simulink library "Renault_Library"  (Simulink 9.2)
settings: Solver=ode3  StartTime=0.0  StopTime=10.0  FixedStep=0.020  RelTol=1e-3  EnableMultiTasking=on
undefined symbols (not defined in the file or in its folder): ABC, AssystemProposal, b, chkval, CoeffA_C, CoeffB_C, CoeffMean_C, ConfTime_C, DiagDelay_C, DividePercent, FailureConfActive_C, FallingEdgeDuration_C, HighLevelThresholdVoltage_C, HighLevelVoltage_C, HighLevelVoltageTunable_C, InitValue_C, InputVoltRangeMax_C, InputVoltRangeMin_C, LowActiveContact_C, LowLevelThresholdVoltage_C, LowLevelVoltage_C, MaxOperatingVoltage_C, MinOperatingVoltage_C, mV2V_conversion, OUT1, OutOfRangeHigh_C, OutOfRangeLow_C, PinSupplySaturationMax, PinSupplySaturationMin, Res1Value_C, Res2Value_C, Res3Value_C, RisingEdgeDuration_C, RSA_CANSignalFailMonitoring, SampleTime_C, service14Table, service19Table, service22Table, service24Table, service28Table, service2ETable, service2FTable, service31Table, service87Table, SizeXStateDID2FTable, SizeXTable14, SizeXTable19, SizeXTable22, SizeXTable24, SizeXTable28, SizeXTable2E, SizeXTable2F, SizeXTable31, SizeXTable87, SizeYStateDID2FTable, SizeYTable14, SizeYTable19, SizeYTable22, SizeYTable24, SizeYTable28 ... (+22)
undefined symbols (not defined in the file): BatteryVoltage_ActivationThreshold_C, FrameStatus_AbsentValue_C, FrameStatus_ConfAbsentValue_C, MemThreshold_C, NAMEOFTHEMODEL_SAMPLE_TIME_CST, NbAbs1_C, NbConfAbs1_C, NbConfPres1_C, NbPres1_C, NOMDUSIGNAL_FaultConfTime_C, NOMDUSIGNAL_FaultRehabTime_C, NOMDUSIGNAL_switchvalue_C, NOMDUSIGNAL_unavailablevalue_C, PeriodFrame1_C, ResetTime_C, WakeUpTime_C
libraries: $bdroot x112, simulink x75
(part 31 of 32)

== stateflow chart Services ECU/Mux/RSA_FrameStatusMgt/FrameMonitoring ==
  state State_Fault_Management_NotAllowed/
    state Frame_present_NA/
      entry:
      Cpt=0;
      during:
      Frame_Status=Frame_Present;
      Cpt=Cpt+Ts;
    state Absent_frame_NA/
      entry: Frame_Status=Frame_Absent_Not_Expected;
    (junction 910) -> Frame_present_NA [order 1] : [Frame_Status==Frame_Present]
    Frame_present_NA -> Frame_present_NA [order 1] : [Frame_Update==1]
    Frame_present_NA -> Absent_frame_NA [order 2] : [(Cpt>=(NbAbs*Period*ToleranceFactor))&&...
        | (Frame_Update==0)]
    Absent_frame_NA -> Frame_present_NA : [Frame_Update==1]
    (default) -> (junction 910)
    (junction 910) -> Absent_frame_NA [order 2] : [Frame_Status~=Frame_Present]
  state State_Fault_Management_Allowed/
    state Frame_present_A/
      entry:
      Cpt=0;
      Frame_Status=Frame_Present;
      during:
      Cpt=Cpt+Ts;
    state Fault_TobeForget_A/
      entry:
      Cpt=0;
      during:
      Cpt=Cpt+Ts;
      Cpt2=Cpt2+Ts;
    state Absent_frame_A/
      entry:
      Frame_Status=Frame_Absent;
      during:
      Cpt=Cpt+Ts;
    state Absent_frame_confirmed_A/
      entry: Cpt2=0;
      Frame_Status=Frame_Confirmed_Absent;
    Frame_present_A -> Frame_present_A [order 1] : [Frame_Update==1]
    Fault_TobeForget_A -> Fault_TobeForget_A [order 1] : [(Frame_Update==1)&&...
        | (Cpt2<NbConfPres*Period*...
        | ToleranceFactor)]
    (default) -> (junction 917)
    (junction 917) -> Frame_present_A [order 1] : [Frame_Status==Frame_Present]
    (junction 917) -> Absent_frame_A [order 2] : [Frame_Status~=Frame_Present]
    Fault_TobeForget_A -> Frame_present_A [order 3] : [(Cpt2>=NbConfPres*Period*ToleranceFactor)]
    Absent_frame_confirmed_A -> Frame_present_A [order 1] : [(Frame_Update==1)&&...
        | NbConfPres==0]
    Frame_present_A -> Absent_frame_A [order 2] : [(Cpt>=(NbAbs*Period*...
        | ToleranceFactor))&&...
        | (Frame_Update==0)]
    Absent_frame_A -> Frame_present_A [order 1] : [Frame_Update==1]
    Fault_TobeForget_A -> Absent_frame_confirmed_A [order 2] : [Cpt>=(NbPres*Period*ToleranceFactor)&&...
        | Frame_Update==0&&NbPres~=0]
    Absent_frame_confirmed_A -> Fault_TobeForget_A [order 2] : [Frame_Update==1&&NbConfPres~=0]
    Absent_frame_A -> Absent_frame_confirmed_A [order 2] : [(Cpt>=(NbConfAbs*Period*ToleranceFactor))&&(Frame_Update==0)]
  (default) -> State_Fault_Management_NotAllowed
  State_Fault_Management_NotAllowed -> State_Fault_Management_Allowed : [ Fault_Management_Allowed == 1 ]
  State_Fault_Management_Allowed -> State_Fault_Management_NotAllowed : [ Fault_Management_Allowed == 0 && ...
      | Frame_Status ~= Frame_Confirmed_Absent ]
  data: Fault_Management_Allowed input boolean; Frame_Update input uint8; Ts input double =Ts; Frame_Status output double =1; Cpt output double; Period input double; NbAbs input double; NbConfAbs input double; NbPres input double; Frame_Absent parameter double; Frame_Absent_Not_Expected parameter double; Frame_Confirmed_Absent parameter double; Frame_Present parameter double; NbConfPres input double; ToleranceFactor parameter double; Cpt2 output double

== stateflow chart Services ECU/PowerSupply/RSA_PowerSupplyMgt/PowerSupMgt_Chart ==
  state Ecu_enabled/
    state WakeUp/
      entry:
      ActivateEcu=Ecu_ON;
      EcuStatus=Status_WAKEUP;
      read = 1;
      write = 0;
    state WithoutInit/
      entry: EcuStatus=Status_ON;
      read = 1;
      write = 0;
    state Ecu_Running/
      entry: EcuStatus=Status_ON;
      read = 0;
      write = 0;
    (default) -> WakeUp [order 1] : [WakeUpTime~=0]
    (default) -> WithoutInit [order 2] : [WakeUpTime==0]
    WakeUp -> Ecu_Running : [after(WakeUpTime/SampleTime,...
        | tick)]
    WithoutInit -> Ecu_Running
  state Ecu_waiting_apc/
    entry: ActivateEcu=Ecu_OFF;
    EcuStatus = Status_OFF;
    read=0;
    write=0;
  state WithoutReset/
    entry: EcuStatus=Status_RESET;
    read = 0;
    write = 1;
  state Reset/
    entry:
    EcuStatus=Status_RESET;
    read = 0;
    write = 1;
  (default) -> Ecu_waiting_apc
  Ecu_waiting_apc -> Ecu_enabled : [Apc==Apc_ON &&...
      | BatteryVoltage>=...
      | BatteryVoltageThreshold]
  Ecu_enabled -> Ecu_waiting_apc [order 2] : [BatteryVoltage<VoltageSupplyCutThreshold]
  Reset -> Ecu_waiting_apc : [after(ResetTime/SampleTime,tick) ||...
      | BatteryVoltage<...
      | VoltageSupplyCutThreshold]
  WithoutReset -> Ecu_waiting_apc
  Ecu_enabled -> WithoutReset [order 3] : [(Apc==Apc_OFF ||...
      | BatteryVoltage<...
      | BatteryVoltageThreshold) &&...
      | ResetTime==0 &&...
      | BatteryVoltage>...
      | VoltageSupplyCutThreshold]
  Ecu_enabled -> Reset [order 1] : [(Apc==Apc_OFF ||...
      | BatteryVoltage<...
      | BatteryVoltageThreshold) &&...
      | ResetTime~=0 &&...
      | BatteryVoltage>...
      | VoltageSupplyCutThreshold]
  data: ActivateEcu output double; Apc input double; BatteryVoltage input double; BatteryVoltageThreshold input double; VoltageSupplyCutThreshold input double; EcuStatus output uint8; read output boolean; WakeUpTime input double; write output boolean; ResetTime input double; Apc_ON parameter double; Apc_OFF parameter double; Ecu_OFF parameter double; Ecu_ON parameter double; Status_OFF parameter double; Status_WAKEUP parameter double; Status_ON parameter double; Status_RESET parameter double; SampleTime input double

== stateflow chart Services ECU/Diag/RSA_DiagnosticManagement_v02.00/DIAGNOSTIC_SANCTION_TOP/Service24/Check_Message_Service24 ==
  (default) -> (junction 996)
  (junction 996) -> (junction 997) [order 1] : [LENGTH_MESSAGE_CORRECT]
  (junction 997) -> (junction 998) [order 1] : [PARAMETER_VALID]
  (junction 998) -> (junction 999) [order 1] : [SECURITY_ACCESS_ACCEPTED]
  (junction 999) -> (junction 1000) [order 1] : [CONDITION_CORRECT]
  (junction 996) -> (junction 1005) [order 2] : {
      | NEGATIVE_RES_CODE= 0x13 ;
      | }
  (junction 997) -> (junction 1001) [order 2] : {
      | NEGATIVE_RES_CODE= 0x31 ;
      | }
  (junction 998) -> (junction 1002) [order 2] : {
      | NEGATIVE_RES_CODE= 0x33 ;
      | }
  (junction 999) -> (junction 1003) [order 2] : {
      | NEGATIVE_RES_CODE= 0x22 ;
      | }
  (junction 1000) -> (junction 1004)
  (junction 1002) -> (junction 1001)
  (junction 1003) -> (junction 1002)
  (junction 1004) -> (junction 1003)
  (junction 1001) -> (junction 1005)
  (junction 1005) -> (junction 1006) : {
      |  SEND_RES_MESSAGE=!SEND_RES_MESSAGE;
      | }
  data: LENGTH_MESSAGE_CORRECT input boolean; CONDITION_CORRECT input boolean; NEGATIVE_RES_CODE output uint8; SECURITY_ACCESS_ACCEPTED input boolean; PARAMETER_VALID input boolean; SEND_RES_MESSAGE output boolean

== stateflow chart Services ECU/Diag/RSA_DiagnosticManagement_v02.00/DIAGNOSTIC_SANCTION_TOP/Service24/Parameter_Valid_Service24/Parameter_service24 ==
  state Session [function]
    (default) -> (junction 1025)
    (junction 1025) -> (junction 1026) [order 1] : [SESSION_ACTIVE==DEFAULT_SESSION]
        | {
        |  s=1;
        | }
    (junction 1025) -> (junction 1027) [order 2]
    (junction 1026) -> (junction 1028)
    (junction 1027) -> (junction 1028) [order 1] : [SESSION_ACTIVE==PROGRAMMING_SESSION]
        | {
        |  s=2;
        | }
    (junction 1027) -> (junction 1029) [order 2]
    (junction 1028) -> (junction 1030)
    (junction 1029) -> (junction 1030) [order 1] : [SESSION_ACTIVE==EXTENDED_SESSION]
        | {
        |  s=3;
        | }
    (junction 1029) -> (junction 1031) [order 2]
    (junction 1030) -> (junction 1032)
    (junction 1031) -> (junction 1032) [order 1] : [SESSION_ACTIVE==SAFETY_SYSTEM_SESSION]
        | {
        |  s=4;
        | }
    (junction 1031) -> (junction 1033) [order 2]
    (junction 1032) -> (junction 1034)
    (junction 1034) -> (junction 1033)
    (junction 1033) -> (junction 1035)
  (default) -> (junction 1061)
  (junction 1061) -> (junction 1064) : {
      |  i=0;...
      |  SECURITY_ACCESS_ACCEPTED=true;...
      |  PARAMETER_VALID= false;...
      |  DID=(DIAG_BUFFER_REQUEST[1]<<8) + DIAG_BUFFER_REQUEST[2];
      | }
  (junction 1062) -> (junction 1063) [order 1] : [DID == service24Table[i][0] &&...
      |  service24Table[i][s]==1]
  (junction 1064) -> (junction 1062) [order 1] : [i<SizeXTable24]
  (junction 1062) -> (junction 1065) [order 2]
  (junction 1063) -> (junction 1067) : {
      |  PARAMETER_VALID= true;
      | }
  (junction 1065) -> (junction 1064) : {
      |  i ++;
      | }
  (junction 1064) -> (junction 1066) [order 2]
  (junction 1067) -> (junction 1066)
  (junction 1066) -> (junction 1068)
  (junction 1068) -> (junction 1069) [order 1] : [service24Table[i][5]==0]
  (junction 1068) -> (junction 1070) [order 2]
  (junction 1069) -> (junction 1071) : {
      |  PARAMETER_VALID=false;
      | }
  (junction 1071) -> (junction 1070)
  (junction 1070) -> (junction 1072)
  data: DIAG_BUFFER_REQUEST input uint8 size=4095; PARAMETER_VALID output boolean; i local uint32; service24Table parameter double size=[SizeXTable24 SizeYTable24]; SizeYTable24 parameter double; SizeXTable24 parameter double; SECURITY_ACCESS_ACCEPTED output boolean; SESSION_ACTIVE input Enum: TYP_SESSION_ACTIVE; s local double; DID local uint16

== stateflow chart Services ECU/Diag/RSA_DiagnosticManagement_v02.00/DIAGNOSTIC_SANCTION_TOP/Service27/Check_Message_Service27 ==
  (default) -> (junction 1105)
  (junction 1099) -> (junction 1100) [order 1] : [SUBFUNCTION_SUPPORTED_IN_ACTIVE_SESSION]
  (junction 1106) -> (junction 1099) [order 1] : [SUBFUNCTION_SUPPORTED]
  (junction 1100) -> (junction 1101) [order 1] : [PARAMETER_VALID]
  (junction 1101) -> (junction 1102) [order 1] : [CONDITION_CORRECT]
  (junction 1102) -> (junction 1103) [order 1] : [REQ_SEQUENCE_OK]
  (junction 1103) -> (junction 1104) [order 1] : [KEY_VALID]
  (junction 1105) -> (junction 1106) [order 1] : [LENGTH_MESSAGE_CORRECT]
  (junction 1099) -> (junction 1109) [order 2] : {
      | NEGATIVE_RES_CODE= 0x7E ;
      | }
  (junction 1100) -> (junction 1110) [order 2] : {
      | NEGATIVE_RES_CODE= 0x31 ;
      | }
  (junction 1101) -> (junction 1111) [order 2] : {
      | NEGATIVE_RES_CODE= 0x22 ;
      | }
  (junction 1102) -> (junction 1112) [order 2] : {
      | NEGATIVE_RES_CODE= 0x24 ;
      | }
  (junction 1103) -> (junction 1113) [order 2] : {
      | NEGATIVE_RES_CODE= 0x35 ;
      | }
  (junction 1104) -> (junction 1114)
  (junction 1105) -> (junction 1107) [order 2] : {
      | NEGATIVE_RES_CODE= 0x13 ;
      | }
  (junction 1106) -> (junction 1108) [order 2] : {
      | NEGATIVE_RES_CODE= 0x12 ;
      | }
  (junction 1108) -> (junction 1107)
  (junction 1109) -> (junction 1108)
  (junction 1110) -> (junction 1109)
  (junction 1111) -> (junction 1110)
  (junction 1112) -> (junction 1111)
  (junction 1113) -> (junction 1112)
  (junction 1114) -> (junction 1113)
  (junction 1107) -> (junction 1115) : {
      |  SEND_RES_MESSAGE=!SEND_RES_MESSAGE;
      | }
  data: LENGTH_MESSAGE_CORRECT input boolean; CONDITION_CORRECT input boolean; REQ_SEQUENCE_OK input boolean; KEY_VALID input double; NEGATIVE_RES_CODE output uint8; PARAMETER_VALID input boolean; SUBFUNCTION_SUPPORTED_IN_ACTIVE_SESSION input boolean; SUBFUNCTION_SUPPORTED input boolean; SEND_RES_MESSAGE output boolean

== stateflow chart Services ECU/Diag/RSA_DiagnosticManagement_v02.00/DIAGNOSTIC_SANCTION_TOP/Service28/Check_Message_Service28 ==
  (default) -> (junction 1153)
  (junction 1149) -> (junction 1150) [order 1] : [SUBFUNCTION_SUPPORTED_IN_ACTIVE_SESSION]
  (junction 1154) -> (junction 1149) [order 1] : [SUBFUNCTION_SUPPORTED]
  (junction 1150) -> (junction 1151) [order 1] : [PARAMETER_VALID]
  (junction 1151) -> (junction 1152) [order 1] : [CONDITION_CORRECT]
  (junction 1153) -> (junction 1154) [order 1] : [LENGTH_MESSAGE_CORRECT]
  (junction 1149) -> (junction 1157) [order 2] : {
      | NEGATIVE_RES_CODE= 0x7E ;
      | }
  (junction 1150) -> (junction 1158) [order 2] : {
      | NEGATIVE_RES_CODE= 0x31 ;
      | }
  (junction 1151) -> (junction 1159) [order 2] : {
      | NEGATIVE_RES_CODE= 0x22 ;
      | }
  (junction 1152) -> (junction 1160)
  (junction 1153) -> (junction 1155) [order 2] : {
      | NEGATIVE_RES_CODE= 0x13 ;
      | }
  (junction 1154) -> (junction 1156) [order 2] : {
      | NEGATIVE_RES_CODE= 0x12 ;
      | }
  (junction 1156) -> (junction 1155)
  (junction 1157) -> (junction 1156)
  (junction 1158) -> (junction 1157)
  (junction 1159) -> (junction 1158)
  (junction 1160) -> (junction 1159)
  (junction 1155) -> (junction 1161) : {
      |  SEND_RES_MESSAGE=!SEND_RES_MESSAGE;
      | }
  data: LENGTH_MESSAGE_CORRECT input boolean; CONDITION_CORRECT input boolean; NEGATIVE_RES_CODE output uint8; SUBFUNCTION_SUPPORTED_IN_ACTIVE_SESSION input boolean; PARAMETER_VALID input boolean; SUBFUNCTION_SUPPORTED input boolean; SEND_RES_MESSAGE output boolean

== stateflow chart Services ECU/Diag/RSA_DiagnosticManagement_v02.00/DIAGNOSTIC_SANCTION_TOP/Service28/Parameter_Valid_Service28/Parameter_service28 ==
  (default) -> (junction 1189)
  (junction 1189) -> (junction 1192) : {
      |  i=0;...
      |  PARAMETER_VALID= false;...
      |  nodeIdentifierData=(DIAG_BUFFER_REQUEST[3]<<8) + DIAG_BUFFER_REQUEST[4];
      | }
  (junction 1190) -> (junction 1191) [order 1] : [nodeIdentifierData == service28Table[i][0]]
  (junction 1192) -> (junction 1190) [order 1] : [i< SizeXTable28]
  (junction 1190) -> (junction 1193) [order 2]
  (junction 1191) -> (junction 1194) : {
      |  PARAMETER_VALID= true;...
      |
      | }
  (junction 1193) -> (junction 1192) : {
      |  i ++;
      | }
  (junction 1192) -> (junction 1195) [order 2]
  (junction 1194) -> (junction 1193)
  data: nodeIdentifierData local uint16; DIAG_BUFFER_REQUEST input uint8 size=4095; PARAMETER_VALID output boolean; i local double; SizeYTable28 parameter double; SizeXTable28 parameter double; service28Table parameter double size=[SizeXTable28 SizeYTable28]

== stateflow chart Services ECU/Diag/RSA_DiagnosticManagement_v02.00/DIAGNOSTIC_SANCTION_TOP/Service2E/Check_Message_Service2E ==
  (default) -> (junction 1214)
  (junction 1214) -> (junction 1215) [order 1] : [LENGTH_MESSAGE_CORRECT]
  (junction 1215) -> (junction 1216) [order 1] : [PARAMETER_VALID]
  (junction 1216) -> (junction 1217) [order 1] : [SECURITY_ACCESS_ACCEPTED]
  (junction 1217) -> (junction 1218) [order 1] : [CONDITION_CORRECT]
  (junction 1218) -> (junction 1219) [order 1] : [CORRECTLY_ALTERED_INTO_MEMORY]
  (junction 1214) -> (junction 1222) [order 2] : {
      | NEGATIVE_RES_CODE= 0x13 ;
      | }
  (junction 1215) -> (junction 1223) [order 2] : {
      | NEGATIVE_RES_CODE= 0x31 ;
      | }
  (junction 1216) -> (junction 1224) [order 2] : {
      | NEGATIVE_RES_CODE= 0x33 ;
      | }
  (junction 1217) -> (junction 1225) [order 2] : {
      | NEGATIVE_RES_CODE= 0x22 ;
      | }
  (junction 1218) -> (junction 1220) [order 2] : {
      | NEGATIVE_RES_CODE= 0x72 ;
      | }
  (junction 1219) -> (junction 1221)
  (junction 1221) -> (junction 1220)
  (junction 1223) -> (junction 1222)
  (junction 1220) -> (junction 1225)
  (junction 1224) -> (junction 1223)
  (junction 1225) -> (junction 1224)
  (junction 1222) -> (junction 1226) : {
      |  SEND_RES_MESSAGE=!SEND_RES_MESSAGE;
      | }
  data: LENGTH_MESSAGE_CORRECT input boolean; CONDITION_CORRECT input boolean; CORRECTLY_ALTERED_INTO_MEMORY input boolean; NEGATIVE_RES_CODE output uint8; SECURITY_ACCESS_ACCEPTED input boolean; PARAMETER_VALID input boolean; SEND_RES_MESSAGE output boolean

== stateflow chart Services ECU/Diag/RSA_DiagnosticManagement_v02.00/DIAGNOSTIC_SANCTION_TOP/Service2E/Parameter_Valid_Service2E/Parameter_service2E ==
  state Session [function]
    (default) -> (junction 1248)
    (junction 1248) -> (junction 1249) [order 1] : [SESSION_ACTIVE==DEFAULT_SESSION]
        | {
        |  s=1;
        | }
    (junction 1248) -> (junction 1250) [order 2]
    (junction 1249) -> (junction 1251)
    (junction 1250) -> (junction 1251) [order 1] : [SESSION_ACTIVE==PROGRAMMING_SESSION]
        | {
        |  s=2;
        | }
    (junction 1250) -> (junction 1252) [order 2]
    (junction 1251) -> (junction 1253)
    (junction 1252) -> (junction 1253) [order 1] : [SESSION_ACTIVE==EXTENDED_SESSION]
        | {
        |  s=3;
        | }
    (junction 1252) -> (junction 1254) [order 2]
    (junction 1253) -> (junction 1255)
    (junction 1254) -> (junction 1255) [order 1] : [SESSION_ACTIVE==SAFETY_SYSTEM_SESSION]
        | {
        |  s=4;
        | }
    (junction 1254) -> (junction 1256) [order 2]
    (junction 1255) -> (junction 1257)
    (junction 1257) -> (junction 1256)
    (junction 1256) -> (junction 1258)
  (default) -> (junction 1288) : {
      |  j=0;...
      |  SECURITY_ACCESS_ACCEPTED=true;...
      |  PARAMETER_VALID= false;...
      |  number_bit_data=0;
      | }
  (junction 1286) -> (junction 1287) [order 1] : [DID == service2ETable[j][0] &&...
      |  service2ETable[j][s]==1  ]
  (junction 1288) -> (junction 1286) [order 1] : [j< SizeXTable2E ]
      | {
      | DID=(DIAG_BUFFER_REQUEST[1]<<8) + DIAG_BUFFER_REQUEST[2];...
      | Session();
      | }
  (junction 1286) -> (junction 1291) [order 2]
  (junction 1287) -> (junction 1289) : {
      |  number_bit_data+=service2ETable[j][6];
      | }
  (junction 1291) -> (junction 1288) : {
      |  j ++;
      | }
  (junction 1288) -> (junction 1297) [order 2]
  (junction 1289) -> (junction 1290) [order 1] : [uint8 ((number_bit_data+7)/8)==(REQ_DATA_NUMBER-3)]
  (junction 1289) -> (junction 1292) [order 2]
  (junction 1290) -> (junction 1293) : {
      |  PARAMETER_VALID= true;
      | }
  (junction 1292) -> (junction 1291)
  (junction 1295) -> (junction 1292)
  (junction 1293) -> (junction 1294) [order 1] : [service2ETable[j][5]==0]
  (junction 1293) -> (junction 1295) [order 2]
  (junction 1294) -> (junction 1296) : {
      |  SECURITY_ACCESS_ACCEPTED=false;
      | }
  (junction 1296) -> (junction 1295)
  data: DIAG_BUFFER_REQUEST input uint8 size=4095; REQ_DATA_NUMBER input uint8; PARAMETER_VALID output boolean; DID local uint16; j local uint32; service2ETable parameter double size=[SizeXTable2E SizeYTable2E]; SizeYTable2E parameter double; SizeXTable2E parameter double; SECURITY_ACCESS_ACCEPTED output boolean; SESSION_ACTIVE input Enum: TYP_SESSION_ACTIVE; s local double; number_bit_data local double

== stateflow chart Services ECU/Diag/RSA_DiagnosticManagement_v02.00/DIAGNOSTIC_SANCTION_TOP/Service2F/Check_Message_Service2F ==
  (default) -> (junction 1323)
  (junction 1323) -> (junction 1322) [order 1] : [LENGTH_MESSAGE_CORRECT]
  (junction 1322) -> (junction 1324) [order 1] : [CONDITION_CORRECT]
  (junction 1324) -> (junction 1325) [order 1] : [PARAMETER_VALID]
  (junction 1325) -> (junction 1326) [order 1] : [SECURITY_ACCESS_ACCEPTED]
  (junction 1322) -> (junction 1327) [order 2] : {
      | NEGATIVE_RES_CODE= 0x22 ;
      | }
  (junction 1323) -> (junction 1328) [order 2] : {
      | NEGATIVE_RES_CODE= 0x13 ;
      | }
  (junction 1324) -> (junction 1329) [order 2] : {
      | NEGATIVE_RES_CODE= 0x31;
      | }
  (junction 1325) -> (junction 1330) [order 2] : {
      | NEGATIVE_RES_CODE= 0x33
      | }
  (junction 1326) -> (junction 1331)
  (junction 1329) -> (junction 1327)
  (junction 1327) -> (junction 1328)
  (junction 1330) -> (junction 1329)
  (junction 1331) -> (junction 1330)
  (junction 1328) -> (junction 1332) : {
      |  SEND_RES_MESSAGE=!SEND_RES_MESSAGE;
      | }
  data: LENGTH_MESSAGE_CORRECT input boolean; CONDITION_CORRECT input boolean; NEGATIVE_RES_CODE output uint8; SECURITY_ACCESS_ACCEPTED input boolean; PARAMETER_VALID input boolean; SEND_RES_MESSAGE output boolean

== stateflow chart Services ECU/Diag/RSA_DiagnosticManagement_v02.00/DIAGNOSTIC_SANCTION_TOP/Service2F/Parameter_Valid_Service2F/Parameter_service2F ==
  state Session [function]
    (default) -> (junction 1351)
    (junction 1351) -> (junction 1352) [order 1] : [SESSION_ACTIVE==DEFAULT_SESSION]
        | {
        |  s=1;
        | }
    (junction 1351) -> (junction 1353) [order 2]
    (junction 1352) -> (junction 1354)
    (junction 1353) -> (junction 1354) [order 1] : [SESSION_ACTIVE==PROGRAMMING_SESSION]
        | {
        |  s=2;
        | }
    (junction 1353) -> (junction 1355) [order 2]
    (junction 1354) -> (junction 1356)
    (junction 1355) -> (junction 1356) [order 1] : [SESSION_ACTIVE==EXTENDED_SESSION]
        | {
        |  s=3;
        | }
    (junction 1355) -> (junction 1357) [order 2]
    (junction 1356) -> (junction 1358)
    (junction 1357) -> (junction 1358) [order 1] : [SESSION_ACTIVE==SAFETY_SYSTEM_SESSION]
        | {
        |  s=4;
        | }
    (junction 1357) -> (junction 1359) [order 2]
    (junction 1358) -> (junction 1360)
    (junction 1360) -> (junction 1359)
    (junction 1359) -> (junction 1361)
  (default) -> (junction 1391)
  (junction 1391) -> (junction 1397) : {
      |  i=0;
      |  j=0;...
      |  PARAMETER_VALID= false;...
      |  DID=(DIAG_BUFFER_REQUEST[1]<<8) + DIAG_BUFFER_REQUEST[2];
      | }
  (junction 1392) -> (junction 1393) [order 1] : [DID== stateDID2FTable[i][0] &&...
      |         DIAG_BUFFER_REQUEST[4]==stateDID2FTable[i][1]]
  (junction 1396) -> (junction 1392) [order 1] : [i<SizeXStateDID2FTable]
  (junction 1392) -> (junction 1400) [order 2]
  (junction 1393) -> (junction 1401) : {
      |  PARAMETER_VALID= true;...
      | }
  (junction 1394) -> (junction 1395) [order 1] : [DID== service2FTable[j][0] &&...
      |         service2FTable[j][s]==1]
  (junction 1395) -> (junction 1396) [order 1] : [DIAG_BUFFER_REQUEST[3]== 3 &&...
      |         service2FTable[j][9]==1 ]
  (junction 1397) -> (junction 1394) [order 1] : [j< SizeXTable2F]
      | {
      |  Session();
      | }
  (junction 1400) -> (junction 1396) : {
      | i++;
      | }
  (junction 1394) -> (junction 1404) [order 2]
  (junction 1395) -> (junction 1398) [order 2]
  (junction 1396) -> (junction 1408) [order 2]
  (junction 1404) -> (junction 1397) : {
      |  j ++;
      | }
  (junction 1397) -> (junction 1411) [order 2]
  (junction 1398) -> (junction 1399) [order 1] : [DIAG_BUFFER_REQUEST[3]== 2 &&...
      |         service2FTable[j][8]==1]
  (junction 1398) -> (junction 1402) [order 2]
  (junction 1399) -> (junction 1407) : {
      |  PARAMETER_VALID= true;...
      | }
  (junction 1401) -> (junction 1400)
  (junction 1402) -> (junction 1403) [order 1] : [DIAG_BUFFER_REQUEST[3]== 1 &&...
      |         service2FTable[j][7]==1]
  (junction 1402) -> (junction 1405) [order 2]
  (junction 1403) -> (junction 1406) : {
      |  PARAMETER_VALID= true;...
      | }
  (junction 1416) -> (junction 1404)
  (junction 1406) -> (junction 1405)
  (junction 1407) -> (junction 1406)
  (junction 1408) -> (junction 1407)
  (junction 1405) -> (junction 1409)
  (junction 1409) -> (junction 1410) [order 1] : [DIAG_BUFFER_REQUEST[3]== 0 &&...
      |         service2FTable[j][6]==1]
  (junction 1409) -> (junction 1412) [order 2]
  (junction 1410) -> (junction 1413) : {
      |  PARAMETER_VALID= true;...
      | }
  (junction 1413) -> (junction 1412)
  (junction 1412) -> (junction 1414)
  (junction 1414) -> (junction 1415) [order 1] : [service2FTable[j][5]==1]
  (junction 1414) -> (junction 1416) [order 2]
  (junction 1415) -> (junction 1417) : {
      |  SECURITY_ACCESS_ACCEPTED=true;
      | }
  (junction 1417) -> (junction 1416)
  data: SESSION_ACTIVE input Enum: TYP_SESSION_ACTIVE; SECURITY_ACCESS_ACCEPTED output double; DIAG_BUFFER_REQUEST input uint8 size=4095; PARAMETER_VALID output boolean; DID local uint16; j local uint32; service2FTable parameter double size=[SizeXTable2F SizeYTable2F]; s local double; SizeYTable2F parameter double; stateDID2FTable parameter double size=[SizeXStateDID2FTable SizeYStateDID2FTable]; SizeXTable2F parameter double; SizeXStateDID2FTable parameter double; SizeYStateDID2FTable parameter double; i local double

== stateflow chart Services ECU/Diag/RSA_DiagnosticManagement_v02.00/DIAGNOSTIC_SANCTION_TOP/Service31/Check_Message_Service31 ==
  (default) -> (junction 1471)
  (junction 1472) -> (junction 1465) [order 1] : [SUBFUNCTION_SUPPORTED]
  (junction 1465) -> (junction 1466) [order 1] : [SUBFUNCTION_SUPPORTED_IN_ACTIVE_SESSION]
  (junction 1466) -> (junction 1467) [order 1] : [PARAMETER_VALID]
  (junction 1467) -> (junction 1468) [order 1] : [SECURITY_ACCESS_ACCEPTED]
  (junction 1468) -> (junction 1469) [order 1] : [CONDITION_CORRECT]
  (junction 1469) -> (junction 1470) [order 1] : [REQ_SEQUENCE_OK]
  (junction 1471) -> (junction 1472) [order 1] : [LENGTH_MESSAGE_CORRECT]
  (junction 1465) -> (junction 1478) [order 2] : {
      | NEGATIVE_RES_CODE= 0x7E ;
      | }
  (junction 1466) -> (junction 1479) [order 2] : {
      | NEGATIVE_RES_CODE= 0x31
      | }
  (junction 1467) -> (junction 1480) [order 2] : {
      | NEGATIVE_RES_CODE= 0x33 ;
      | }
  (junction 1468) -> (junction 1474) [order 2] : {
      | NEGATIVE_RES_CODE= 0x22 ;
      | }
  (junction 1469) -> (junction 1475) [order 2] : {
      | NEGATIVE_RES_CODE= 0x24 ;
      | }
  (junction 1470) -> (junction 1476)
  (junction 1471) -> (junction 1473) [order 2] : {
      | NEGATIVE_RES_CODE= 0x13 ;
      | }
  (junction 1472) -> (junction 1477) [order 2] : {
      | NEGATIVE_RES_CODE= 0x12 ;
      | }
  (junction 1477) -> (junction 1473)
  (junction 1475) -> (junction 1474)
  (junction 1476) -> (junction 1475)
  (junction 1474) -> (junction 1480)
  (junction 1478) -> (junction 1477)
  (junction 1479) -> (junction 1478)
  (junction 1480) -> (junction 1479)
  (junction 1473) -> (junction 1481) : {
      |  SEND_RES_MESSAGE=!SEND_RES_MESSAGE;
      | }
  data: LENGTH_MESSAGE_CORRECT input boolean; REQ_SEQUENCE_OK input boolean; NEGATIVE_RES_CODE output uint8; CONDITION_CORRECT input boolean; SECURITY_ACCESS_ACCEPTED input boolean; PARAMETER_VALID input double; SUBFUNCTION_SUPPORTED_IN_ACTIVE_SESSION input boolean; SUBFUNCTION_SUPPORTED input boolean; SEND_RES_MESSAGE output boolean

== stateflow chart Services ECU/Diag/RSA_DiagnosticManagement/DIAGNOSTIC_SANCTION_TOP/Service10/Check_Message_Service10 ==
  (default) -> (junction 1513)
  (junction 1513) -> (junction 1514) [order 1] : [LENGTH_MESSAGE_CORRECT]
  (junction 1514) -> (junction 1515) [order 1] : [SUBFUNCTION_SUPPORTED]
  (junction 1515) -> (junction 1516) [order 1] : [CONDITION_CORRECT]
  (junction 1513) -> (junction 1517) [order 2] : {
      | NEGATIVE_RES_CODE= 0x13 ;
      | }
  (junction 1514) -> (junction 1518) [order 2] : {
      | NEGATIVE_RES_CODE= 0x12 ;
      | }
  (junction 1515) -> (junction 1519) [order 2] : {
      | NEGATIVE_RES_CODE= 0x22 ;
      | }
  (junction 1516) -> (junction 1520)
  (junction 1518) -> (junction 1517)
  (junction 1519) -> (junction 1518)
  (junction 1520) -> (junction 1519)
  (junction 1517) -> (junction 1521) : {
      |  SEND_RES_MESSAGE=!SEND_RES_MESSAGE;
      | }
  data: SUBFUNCTION_SUPPORTED input boolean; LENGTH_MESSAGE_CORRECT input boolean; CONDITION_CORRECT input boolean; NEGATIVE_RES_CODE output uint8; SEND_RES_MESSAGE output boolean

== stateflow chart Services ECU/Diag/RSA_DiagnosticManagement/DIAGNOSTIC_SANCTION_TOP/Service11/Check_Message_Service11 ==
  (default) -> (junction 1548)
  (junction 1543) -> (junction 1544) [order 1] : [SUBFUNCTION_SUPPORTED_IN_ACTIVE_SESSION]
  (junction 1544) -> (junction 1545) [order 1] : [SECURITY_ACCESS_ACCEPTED]
  (junction 1545) -> (junction 1546) [order 1] : [CONDITION_CORRECT]
  (junction 1547) -> (junction 1543) [order 1] : [SUBFUNCTION_SUPPORTED]
  (junction 1548) -> (junction 1547) [order 1] : [LENGTH_MESSAGE_CORRECT]
  (junction 1543) -> (junction 1551) [order 2] : {
      | NEGATIVE_RES_CODE= 0x7E ;
      | }
  (junction 1544) -> (junction 1552) [order 2] : {
      | NEGATIVE_RES_CODE= 0x33 ;
      | }
  (junction 1545) -> (junction 1553) [order 2] : {
      | NEGATIVE_RES_CODE= 0x22 ;
      | }
  (junction 1546) -> (junction 1554)
  (junction 1547) -> (junction 1550) [order 2] : {
      | NEGATIVE_RES_CODE= 0x12 ;
      | }
  (junction 1548) -> (junction 1549) [order 2] : {
      | NEGATIVE_RES_CODE= 0x13 ;
      | }
  (junction 1550) -> (junction 1549)
  (junction 1551) -> (junction 1550)
  (junction 1552) -> (junction 1551)
  (junction 1553) -> (junction 1552)
  (junction 1554) -> (junction 1553)
  (junction 1549) -> (junction 1555) : {
      |  SEND_RES_MESSAGE=!SEND_RES_MESSAGE;
      | }
  data: SUBFUNCTION_SUPPORTED input boolean; SUBFUNCTION_SUPPORTED_IN_ACTIVE_SESSION input boolean; LENGTH_MESSAGE_CORRECT input boolean; CONDITION_CORRECT input boolean; NEGATIVE_RES_CODE output uint8; SECURITY_ACCESS_ACCEPTED input boolean; SEND_RES_MESSAGE output boolean

== stateflow chart Services ECU/Diag/RSA_DiagnosticManagement/DIAGNOSTIC_SANCTION_TOP/Service14/Check_Message_Service14 ==
  (default) -> (junction 1584)
  (junction 1581) -> (junction 1582) [order 1] : [PARAMETER_VALID]
  (junction 1582) -> (junction 1583) [order 1] : [CONDITION_CORRECT]
  (junction 1584) -> (junction 1581) [order 1] : [LENGTH_MESSAGE_CORRECT]
  (junction 1581) -> (junction 1585) [order 2] : {
      | NEGATIVE_RES_CODE= 0x31 ;
      | }
  (junction 1582) -> (junction 1586) [order 2] : {
      | NEGATIVE_RES_CODE= 0x22 ;
      | }
  (junction 1583) -> (junction 1587)
  (junction 1584) -> (junction 1588) [order 2] : {
      | NEGATIVE_RES_CODE= 0x13 ;
      | }
  (junction 1586) -> (junction 1585)
  (junction 1587) -> (junction 1586)
  (junction 1585) -> (junction 1588)
  (junction 1588) -> (junction 1589) : {
      |  SEND_RES_MESSAGE=!SEND_RES_MESSAGE;
      | }
  data: LENGTH_MESSAGE_CORRECT input boolean; CONDITION_CORRECT input boolean; NEGATIVE_RES_CODE output uint8; PARAMETER_VALID input boolean; SEND_RES_MESSAGE output boolean

== stateflow chart Services ECU/Diag/RSA_DiagnosticManagement/DIAGNOSTIC_SANCTION_TOP/Service14/Parameter_Valid_Service14/Parameter_service14 ==
  state Session [function]
    (default) -> (junction 1605)
    (junction 1605) -> (junction 1606) [order 1] : [SESSION_ACTIVE==DEFAULT_SESSION]
        | {
        |  s=1;
        | }
    (junction 1605) -> (junction 1607) [order 2]
    (junction 1606) -> (junction 1608)
    (junction 1607) -> (junction 1608) [order 1] : [SESSION_ACTIVE==PROGRAMMING_SESSION]
        | {
        |  s=2;
        | }
    (junction 1607) -> (junction 1609) [order 2]
    (junction 1608) -> (junction 1610)
    (junction 1609) -> (junction 1610) [order 1] : [SESSION_ACTIVE==EXTENDED_SESSION]
        | {
        |  s=3;
        | }
    (junction 1609) -> (junction 1611) [order 2]
    (junction 1610) -> (junction 1612)
    (junction 1611) -> (junction 1612) [order 1] : [SESSION_ACTIVE==SAFETY_SYSTEM_SESSION]
        | {
        |  s=4;
        | }
    (junction 1611) -> (junction 1613) [order 2]
    (junction 1612) -> (junction 1614)
    (junction 1614) -> (junction 1613)
    (junction 1613) -> (junction 1615)
  (default) -> (junction 1641) : {
      |  j=0;...
      |  PARAMETER_VALID= false;...
      | }
  (junction 1639) -> (junction 1640) [order 1] : [DIAG_BUFFER_REQUEST[1] == service14Table[j][0] &&...
      |    DIAG_BUFFER_REQUEST[2] == service14Table[j][1] &&...
      |    DIAG_BUFFER_REQUEST[3] == service14Table[j][2] &&...
      |         service14Table[j][s+2]==1]
  (junction 1641) -> (junction 1639) [order 1] : [j< SizeXTable14 ]
      | {
      |  Session();
      | }
  (junction 1639) -> (junction 1642) [order 2]
  (junction 1640) -> (junction 1643) : {
      |  PARAMETER_VALID= true;...
      | }
  (junction 1642) -> (junction 1641) : {
      |  j ++;
      | }
  (junction 1641) -> (junction 1644) [order 2]
  (junction 1643) -> (junction 1642)
  data: DIAG_BUFFER_REQUEST input uint8 size=4095; PARAMETER_VALID output boolean; j local uint32; service14Table parameter double size=[SizeXTable14 SizeYTable14]; SizeYTable14 parameter double; SizeXTable14 parameter double; SESSION_ACTIVE input Enum: TYP_SESSION_ACTIVE; s local double

== stateflow chart Services ECU/Diag/RSA_DiagnosticManagement/DIAGNOSTIC_SANCTION_TOP/Service19/Check_Message_Service19 ==
  (default) -> (junction 1664)
  (junction 1661) -> (junction 1662) [order 1] : [SUBFUNCTION_SUPPORTED_IN_ACTIVE_SESSION]
  (junction 1662) -> (junction 1663) [order 1] : [PARAMETER_VALID]
  (junction 1665) -> (junction 1661) [order 1] : [SUBFUNCTION_SUPPORTED]
  (junction 1664) -> (junction 1665) [order 1] : [LENGTH_MESSAGE_CORRECT]
  (junction 1661) -> (junction 1668) [order 2] : {
      | NEGATIVE_RES_CODE= 0x7E ;
      | }
  (junction 1662) -> (junction 1669) [order 2] : {
      | NEGATIVE_RES_CODE= 0x31 ;
      | }
  (junction 1663) -> (junction 1670)
  (junction 1664) -> (junction 1666) [order 2] : {
      | NEGATIVE_RES_CODE= 0x13 ;
      | }
  (junction 1665) -> (junction 1667) [order 2] : {
      | NEGATIVE_RES_CODE= 0x12 ;
      | }
  (junction 1667) -> (junction 1666)
  (junction 1668) -> (junction 1667)
  (junction 1669) -> (junction 1668)
  (junction 1670) -> (junction 1669)
  (junction 1666) -> (junction 1671) : {
      |  SEND_RES_MESSAGE=!SEND_RES_MESSAGE;
      | }
  data: LENGTH_MESSAGE_CORRECT input boolean; NEGATIVE_RES_CODE output uint8; SUBFUNCTION_SUPPORTED_IN_ACTIVE_SESSION input boolean; PARAMETER_VALID input boolean; SUBFUNCTION_SUPPORTED input boolean; SEND_RES_MESSAGE output boolean

== stateflow chart Services ECU/Diag/RSA_DiagnosticManagement/DIAGNOSTIC_SANCTION_TOP/Service19/Parameter_Valid_Service19/Parameter_service19 ==
  (default) -> (junction 1695) : {
      |  j=0;...
      |  PARAMETER_VALID= false;
      | }
  (junction 1695) -> (junction 1696) [order 1] : [DIAG_BUFFER_REQUEST[1]==0x01 ||  DIAG_BUFFER_REQUEST[1]==0x11 ||...
      |    DIAG_BUFFER_REQUEST[1]==0x12 ||  DIAG_BUFFER_REQUEST[1]==0x02 ||...
      |    DIAG_BUFFER_REQUEST[1]==0x0F ||  DIAG_BUFFER_REQUEST[1]==0x13 ||...
      |    DIAG_BUFFER_REQUEST[1]==0x03 ||  DIAG_BUFFER_REQUEST[1]==0x07 ||...
      |    DIAG_BUFFER_REQUEST[1]==0x08 ||  DIAG_BUFFER_REQUEST[1]==0x13 ||...
      |    DIAG_BUFFER_REQUEST[1]==0x0A ||  DIAG_BUFFER_REQUEST[1]==0x0B ||...
      |    DIAG_BUFFER_REQUEST[1]==0x0C ||  DIAG_BUFFER_REQUEST[1]==0x0D ||...
      |    DIAG_BUFFER_REQUEST[1]==0x0E || DIAG_BUFFER_REQUEST[1]==0x14 ||...
      |                                                              DIAG_BUFFER_REQUEST[1]==0x15]
  (junction 1695) -> (junction 1697) [order 2]
  (junction 1696) -> (junction 1698) : {
      |  PARAMETER_VALID= true;...
      | }
  (junction 1698) -> (junction 1697)
  (junction 1697) -> (junction 1702)
  (junction 1699) -> (junction 1700) [order 1] : [DIAG_BUFFER_REQUEST[2]== service19Table[j][0] &&...
      |         DIAG_BUFFER_REQUEST[3]== service19Table[j][1] &&...
      |        DIAG_BUFFER_REQUEST[4]== service19Table[j][2] ]
  (junction 1700) -> (junction 1701) [order 1] : [DIAG_BUFFER_REQUEST[1]==0x04 &&...
      |     DIAG_BUFFER_REQUEST[5]==service19Table[j][3]]
  (junction 1703) -> (junction 1699) [order 1] : [j< SizeXTable19 ]
  (junction 1699) -> (junction 1706) [order 2]
  (junction 1700) -> (junction 1704) [order 2]
  (junction 1701) -> (junction 1714) : {
      |  PARAMETER_VALID= true;
      | }
  (junction 1702) -> (junction 1703) [order 1] : [DIAG_BUFFER_REQUEST[1]==0x04 ||  DIAG_BUFFER_REQUEST[1]==0x09 ||...
      |    DIAG_BUFFER_REQUEST[1]==0x10 ||  DIAG_BUFFER_REQUEST[1]==0x06]
  (junction 1706) -> (junction 1703) : {
      |  j ++;
      | }
  (junction 1702) -> (junction 1709) [order 2]
  (junction 1703) -> (junction 1710) [order 2]
  (junction 1704) -> (junction 1705) [order 1] : [(DIAG_BUFFER_REQUEST[1]==0x06 ||...
      |    DIAG_BUFFER_REQUEST[1]==0x10) &&...
      |     DIAG_BUFFER_REQUEST[5]==0xFF]
  (junction 1704) -> (junction 1707) [order 2]
  (junction 1705) -> (junction 1713) : {
      |  PARAMETER_VALID= true;
      | }
  (junction 1707) -> (junction 1708) [order 1] : [DIAG_BUFFER_REQUEST[1]==0x09]
  (junction 1711) -> (junction 1706)
  (junction 1710) -> (junction 1709)
  (junction 1707) -> (junction 1711) [order 2]
  (junction 1708) -> (junction 1712) : {
      |  PARAMETER_VALID= true;
      | }
  (junction 1709) -> (junction 1715)
  (junction 1712) -> (junction 1711)
  (junction 1713) -> (junction 1712)
  (junction 1714) -> (junction 1713)
  data: DIAG_BUFFER_REQUEST input uint8 size=4095; PARAMETER_VALID output boolean; j local uint32; service19Table parameter double size=[SizeXTable19 SizeYTable19]; SizeXTable19 parameter double; SizeYTable19 parameter double

== stateflow chart Services ECU/Diag/RSA_DiagnosticManagement/DIAGNOSTIC_SANCTION_TOP/Service22/Check_Message_Service22 ==
  (default) -> (junction 1758)
  (junction 1753) -> (junction 1754) [order 1] : [PARAMETER_VALID]
  (junction 1754) -> (junction 1755) [order 1] : [SECURITY_ACCESS_ACCEPTED]
  (junction 1755) -> (junction 1756) [order 1] : [CONDITION_CORRECT]
  (junction 1756) -> (junction 1757) [order 1] : [LENGTH_RESPONSE_CORRECT]
  (junction 1758) -> (junction 1753) [order 1] : [LENGTH_MESSAGE_CORRECT]
  (junction 1753) -> (junction 1759) [order 2] : {
      | NEGATIVE_RES_CODE= 0x31 ;
      | }
  (junction 1754) -> (junction 1760) [order 2] : {
      | NEGATIVE_RES_CODE= 0x33 ;
      | }
  (junction 1755) -> (junction 1761) [order 2] : {
      | NEGATIVE_RES_CODE= 0x22 ;
      | }
  (junction 1756) -> (junction 1762) [order 2] : {
      | NEGATIVE_RES_CODE= 0x14 ;
      | }
  (junction 1757) -> (junction 1763)
  (junction 1758) -> (junction 1764) [order 2] : {
      | NEGATIVE_RES_CODE= 0x13 ;
      | }
  (junction 1760) -> (junction 1759)
  (junction 1761) -> (junction 1760)
  (junction 1762) -> (junction 1761)
  (junction 1763) -> (junction 1762)
  (junction 1759) -> (junction 1764)
  (junction 1764) -> (junction 1765) : {
      |  SEND_RES_MESSAGE=!SEND_RES_MESSAGE;
      | }
  data: LENGTH_MESSAGE_CORRECT input boolean; CONDITION_CORRECT input boolean; NEGATIVE_RES_CODE output uint8; LENGTH_RESPONSE_CORRECT input boolean; SECURITY_ACCESS_ACCEPTED input boolean; PARAMETER_VALID input boolean; SEND_RES_MESSAGE output boolean

== stateflow chart Services ECU/Diag/RSA_DiagnosticManagement/DIAGNOSTIC_SANCTION_TOP/Service22/Parameter_Valid_Service22/Parameter_service22 ==
  state Session [function]
    (default) -> (junction 1788)
    (junction 1788) -> (junction 1789) [order 1] : [SESSION_ACTIVE==DEFAULT_SESSION]
        | {
        |  s=1;
        | }
    (junction 1788) -> (junction 1790) [order 2]
    (junction 1789) -> (junction 1791)
    (junction 1790) -> (junction 1791) [order 1] : [SESSION_ACTIVE==PROGRAMMING_SESSION]
        | {
        |  s=2;
        | }
    (junction 1790) -> (junction 1792) [order 2]
    (junction 1791) -> (junction 1793)
    (junction 1792) -> (junction 1793) [order 1] : [SESSION_ACTIVE==EXTENDED_SESSION]
        | {
        |  s=3;
        | }
    (junction 1792) -> (junction 1794) [order 2]
    (junction 1793) -> (junction 1795)
    (junction 1794) -> (junction 1795) [order 1] : [SESSION_ACTIVE==SAFETY_SYSTEM_SESSION]
        | {
        |  s=4;
        | }
    (junction 1794) -> (junction 1796) [order 2]
    (junction 1795) -> (junction 1797)
    (junction 1797) -> (junction 1796)
    (junction 1796) -> (junction 1798)
  state Max_data_size_bit [function]
    (default) -> (junction 1814)
    (junction 1814) -> (junction 1815) [order 1] : [DATA_SIZE_BIT < (service22Table[j][6]+service22Table[j][7]-1)]
    (junction 1814) -> (junction 1816) [order 2]
    (junction 1815) -> (junction 1817) : {
        |  DATA_SIZE_BIT=(service22Table[j][6]+service22Table[j][7]-1);
        | }
    (junction 1817) -> (junction 1816)
    (junction 1816) -> (junction 1818)
  (default) -> (junction 1844) : {
      |  i=0;...
      |  j=0;...
      |  w=0;...
      |  SECURITY_ACCESS_ACCEPTED=true;...
      |  PARAMETER_VALID= false;...
      |  DATA_SIZE_BIT=0;...
      |  LIST_DID_SERVICE_22=0;...
      |  RESPONSE_SIZE=2;
      | }
  (junction 1844) -> (junction 1841) [order 1] : [i<((REQ_DATA_NUMBER-1))]
  (junction 1841) -> (junction 1849) : {
      |  DID=(DIAG_BUFFER_REQUEST[i+1]<<8) + DIAG_BUFFER_REQUEST[i+2];
      | }
  (junction 1842) -> (junction 1843) [order 1] : [DID == service22Table[j][0] &&...
      |  service22Table[j][s]==1]
  (junction 1846) -> (junction 1842) [order 1] : [j< SizeXTable22 ]
  (junction 1842) -> (junction 1850) [order 2]
  (junction 1843) -> (junction 1847) : {
      |  Max_data_size_bit();
      | }
  (junction 1849) -> (junction 1844) [order 2] : {
      |  i =i+2;...
      |  DATA_SIZE_BIT=0;...
      |  j=0;
      | }
  (junction 1844) -> (junction 1855) [order 2]
  (junction 1845) -> (junction 1846) [order 1] : [DID == service22Table[j][0] &&...
      |  service22Table[j][s]==1]
  (junction 1847) -> (junction 1848) [order 1] : [service22Table[j][5]==0]
  (junction 1849) -> (junction 1845) [order 1] : [j< SizeXTable22]
      | {
      |  Session();
      | }
  (junction 1850) -> (junction 1846) : {
      |  j ++;
      | }
  (junction 1845) -> (junction 1853) [order 2]
  (junction 1846) -> (junction 1854) [order 2] : {
      |  PARAMETER_VALID= true;...
      |  LIST_DID_SERVICE_22[w][0] = DID;...
      |  LIST_DID_SERVICE_22[w][1] = RESPONSE_SIZE-1;...
      |  LIST_DID_SERVICE_22[w][2] =(DATA_SIZE_BIT+7)/8-3;...
      |  w ++;...
      |  RESPONSE_SIZE=RESPONSE_SIZE+((DATA_SIZE_BIT+7)/8)-1 ;
      | }
  (junction 1847) -> (junction 1851) [order 2]
  (junction 1848) -> (junction 1852) : {
      |  SECURITY_ACCESS_ACCEPTED=false;
      | }
  (junction 1853) -> (junction 1849) : {
      |  j ++;
      | }
  (junction 1851) -> (junction 1850)
  (junction 1852) -> (junction 1851)
  (junction 1854) -> (junction 1853)
  data: DIAG_BUFFER_REQUEST input uint8 size=4095; REQ_DATA_NUMBER input uint8; PARAMETER_VALID output boolean; LIST_DID_SERVICE_22 output uint16 size=[2046 3]; DID local uint16; w local uint32; i local uint32; j local uint32; service22Table parameter double size=[SizeXTable22 SizeYTable22]; SizeYTable22 parameter double; SizeXTable22 parameter double; RESPONSE_SIZE output uint16; SECURITY_ACCESS_ACCEPTED output boolean; SESSION_ACTIVE input Enum: TYP_SESSION_ACTIVE; s local double; DATA_SIZE_BIT local double

== stateflow chart Services ECU/Diag/RSA_DiagnosticManagement/DIAGNOSTIC_SANCTION_TOP/Service23/Check_Message_Service23 ==
  (default) -> (junction 1885)
  (junction 1885) -> (junction 1886) [order 1] : [LENGTH_MESSAGE_CORRECT]
  (junction 1886) -> (junction 1887) [order 1] : [PARAMETER_VALID]
  (junction 1887) -> (junction 1888) [order 1] : [SECURITY_ACCESS_ACCEPTED]
  (junction 1888) -> (junction 1889) [order 1] : [CONDITION_CORRECT]
  (junction 1885) -> (junction 1894) [order 2] : {
      | NEGATIVE_RES_CODE= 0x13 ;
      | }
  (junction 1886) -> (junction 1890) [order 2] : {
      | NEGATIVE_RES_CODE= 0x31 ;
      | }
  (junction 1887) -> (junction 1891) [order 2] : {
      | NEGATIVE_RES_CODE= 0x33 ;
      | }
  (junction 1888) -> (junction 1892) [order 2] : {
      | NEGATIVE_RES_CODE= 0x22 ;
      | }
  (junction 1889) -> (junction 1893)
  (junction 1891) -> (junction 1890)
  (junction 1892) -> (junction 1891)
  (junction 1893) -> (junction 1892)
  (junction 1890) -> (junction 1894)
  (junction 1894) -> (junction 1895) : {
      |  SEND_RES_MESSAGE=!SEND_RES_MESSAGE;
      | }
  data: LENGTH_MESSAGE_CORRECT input boolean; CONDITION_CORRECT input boolean; NEGATIVE_RES_CODE output uint8; SECURITY_ACCESS_ACCEPTED input boolean; PARAMETER_VALID input boolean; SEND_RES_MESSAGE output boolean

== stateflow chart Services ECU/Diag/RSA_DiagnosticManagement/DIAGNOSTIC_SANCTION_TOP/Service23/Parameter_Valid_Service23/Parameter_service23 ==
  state Session [function]
    (default) -> (junction 1914)
    (junction 1914) -> (junction 1915) [order 1] : [SESSION_ACTIVE==DEFAULT_SESSION]
        | {
        |  s=1;
        | }
    (junction 1914) -> (junction 1916) [order 2]
    (junction 1915) -> (junction 1917)
    (junction 1916) -> (junction 1917) [order 1] : [SESSION_ACTIVE==PROGRAMMING_SESSION]
        | {
        |  s=2;
        | }
    (junction 1916) -> (junction 1918) [order 2]
    (junction 1917) -> (junction 1919)
    (junction 1918) -> (junction 1919) [order 1] : [SESSION_ACTIVE==EXTENDED_SESSION]
        | {
        |  s=3;
        | }
    (junction 1918) -> (junction 1920) [order 2]
    (junction 1919) -> (junction 1921)
    (junction 1920) -> (junction 1921) [order 1] : [SESSION_ACTIVE==SAFETY_SYSTEM_SESSION]
        | {
        |  s=4;
        | }
    (junction 1920) -> (junction 1922) [order 2]
    (junction 1921) -> (junction 1923)
    (junction 1923) -> (junction 1922)
    (junction 1922) -> (junction 1924)
  (default) -> (junction 1949) : {
      |  i=1;...
      |  SECURITY_ACCESS_ACCEPTED=false;...
      |  PARAMETER_VALID= false;...
      |  memorySize=0;
      | }
  (junction 1949) -> (junction 1948) [order 1] : [i<=(DIAG_BUFFER_REQUEST[1]&0x0F)]
  (junction 1948) -> (junction 1950) : {
      |  memorySize+=DIAG_BUFFER_REQUEST[REQ_DATA_NUMBER-i]<<((i-1)*8);
      | }
  (junction 1950) -> (junction 1949) : {
      |  i ++;
      | }
  (junction 1949) -> (junction 1951) [order 2]
  (junction 1951) -> (junction 1952) [order 1] : [memorySize!=0 && ...
      |  memorySize<4095]
  (junction 1951) -> (junction 1953) [order 2]
  (junction 1952) -> (junction 1954) : {
      |  PARAMETER_VALID=true;
      | }
  (junction 1954) -> (junction 1953)
  (junction 1953) -> (junction 1955)
  data: DIAG_BUFFER_REQUEST input uint8 size=4095; REQ_DATA_NUMBER input uint8; PARAMETER_VALID output boolean; i local uint32; SECURITY_ACCESS_ACCEPTED output boolean; SESSION_ACTIVE input Enum: TYP_SESSION_ACTIVE; s local double; memorySize local double

== stateflow chart Services ECU/Diag/RSA_DiagnosticManagement/DIAGNOSTIC_SANCTION_TOP/Service24/Check_Message_Service24 ==
  (default) -> (junction 1974)
  (junction 1974) -> (junction 1975) [order 1] : [LENGTH_MESSAGE_CORRECT]
  (junction 1975) -> (junction 1976) [order 1] : [PARAMETER_VALID]
  (junction 1976) -> (junction 1977) [order 1] : [SECURITY_ACCESS_ACCEPTED]
  (junction 1977) -> (junction 1978) [order 1] : [CONDITION_CORRECT]
  (junction 1974) -> (junction 1983) [order 2] : {
      | NEGATIVE_RES_CODE= 0x13 ;
      | }
  (junction 1975) -> (junction 1979) [order 2] : {
      | NEGATIVE_RES_CODE= 0x31 ;
      | }
  (junction 1976) -> (junction 1980) [order 2] : {
      | NEGATIVE_RES_CODE= 0x33 ;
      | }
  (junction 1977) -> (junction 1981) [order 2] : {
      | NEGATIVE_RES_CODE= 0x22 ;
      | }
  (junction 1978) -> (junction 1982)
  (junction 1980) -> (junction 1979)
  (junction 1981) -> (junction 1980)
  (junction 1982) -> (junction 1981)
  (junction 1979) -> (junction 1983)
  (junction 1983) -> (junction 1984) : {
      |  SEND_RES_MESSAGE=!SEND_RES_MESSAGE;
      | }
  data: LENGTH_MESSAGE_CORRECT input boolean; CONDITION_CORRECT input boolean; NEGATIVE_RES_CODE output uint8; SECURITY_ACCESS_ACCEPTED input boolean; PARAMETER_VALID input boolean; SEND_RES_MESSAGE output boolean

== stateflow chart Services ECU/Diag/RSA_DiagnosticManagement/DIAGNOSTIC_SANCTION_TOP/Service24/Parameter_Valid_Service24/Parameter_service24 ==
  state Session [function]
    (default) -> (junction 2003)
    (junction 2003) -> (junction 2004) [order 1] : [SESSION_ACTIVE==DEFAULT_SESSION]
        | {
        |  s=1;
        | }
    (junction 2003) -> (junction 2005) [order 2]
    (junction 2004) -> (junction 2006)
    (junction 2005) -> (junction 2006) [order 1] : [SESSION_ACTIVE==PROGRAMMING_SESSION]
        | {
        |  s=2;
        | }
    (junction 2005) -> (junction 2007) [order 2]
    (junction 2006) -> (junction 2008)
    (junction 2007) -> (junction 2008) [order 1] : [SESSION_ACTIVE==EXTENDED_SESSION]
        | {
        |  s=3;
        | }
    (junction 2007) -> (junction 2009) [order 2]
    (junction 2008) -> (junction 2010)
    (junction 2009) -> (junction 2010) [order 1] : [SESSION_ACTIVE==SAFETY_SYSTEM_SESSION]
        | {
        |  s=4;
        | }
    (junction 2009) -> (junction 2011) [order 2]
    (junction 2010) -> (junction 2012)
    (junction 2012) -> (junction 2011)
    (junction 2011) -> (junction 2013)
  (default) -> (junction 2039)
  (junction 2039) -> (junction 2042) : {
      |  i=0;...
      |  SECURITY_ACCESS_ACCEPTED=true;...
      |  PARAMETER_VALID= false;...
      |  DID=(DIAG_BUFFER_REQUEST[1]<<8) + DIAG_BUFFER_REQUEST[2];
      | }
  (junction 2040) -> (junction 2041) [order 1] : [DID == service24Table[i][0] &&...
      |  service24Table[i][s]==1]
  (junction 2042) -> (junction 2040) [order 1] : [i<SizeXTable24]
  (junction 2040) -> (junction 2043) [order 2]
  (junction 2041) -> (junction 2045) : {
      |  PARAMETER_VALID= true;
      | }
  (junction 2043) -> (junction 2042) : {
      |  i ++;
      | }
  (junction 2042) -> (junction 2044) [order 2]
  (junction 2045) -> (junction 2044)
  (junction 2044) -> (junction 2046)
  (junction 2046) -> (junction 2047) [order 1] : [service24Table[i][5]==0]
  (junction 2046) -> (junction 2048) [order 2]
  (junction 2047) -> (junction 2049) : {
      |  PARAMETER_VALID=false;
      | }
  (junction 2049) -> (junction 2048)
  (junction 2048) -> (junction 2050)
  data: DIAG_BUFFER_REQUEST input uint8 size=4095; PARAMETER_VALID output boolean; i local uint32; service24Table parameter double size=[SizeXTable24 SizeYTable24]; SizeYTable24 parameter double; SizeXTable24 parameter double; SECURITY_ACCESS_ACCEPTED output boolean; SESSION_ACTIVE input Enum: TYP_SESSION_ACTIVE; s local double; DID local uint16

== stateflow chart Services ECU/Diag/RSA_DiagnosticManagement/DIAGNOSTIC_SANCTION_TOP/Service27/Check_Message_Service27 ==
  (default) -> (junction 2083)
  (junction 2077) -> (junction 2078) [order 1] : [SUBFUNCTION_SUPPORTED_IN_ACTIVE_SESSION]
  (junction 2084) -> (junction 2077) [order 1] : [SUBFUNCTION_SUPPORTED]
  (junction 2078) -> (junction 2079) [order 1] : [PARAMETER_VALID]
  (junction 2079) -> (junction 2080) [order 1] : [CONDITION_CORRECT]
  (junction 2080) -> (junction 2081) [order 1] : [REQ_SEQUENCE_OK]
  (junction 2081) -> (junction 2082) [order 1] : [KEY_VALID]
  (junction 2083) -> (junction 2084) [order 1] : [LENGTH_MESSAGE_CORRECT]
  (junction 2077) -> (junction 2087) [order 2] : {
      | NEGATIVE_RES_CODE= 0x7E ;
      | }
  (junction 2078) -> (junction 2088) [order 2] : {
      | NEGATIVE_RES_CODE= 0x31 ;
      | }
  (junction 2079) -> (junction 2089) [order 2] : {
      | NEGATIVE_RES_CODE= 0x22 ;
      | }
  (junction 2080) -> (junction 2090) [order 2] : {
      | NEGATIVE_RES_CODE= 0x24 ;
      | }
  (junction 2081) -> (junction 2091) [order 2] : {
      | NEGATIVE_RES_CODE= 0x35 ;
      | }
  (junction 2082) -> (junction 2092)
  (junction 2083) -> (junction 2085) [order 2] : {
      | NEGATIVE_RES_CODE= 0x13 ;
      | }
  (junction 2084) -> (junction 2086) [order 2] : {
      | NEGATIVE_RES_CODE= 0x12 ;
      | }
  (junction 2086) -> (junction 2085)
  (junction 2087) -> (junction 2086)
  (junction 2088) -> (junction 2087)
  (junction 2089) -> (junction 2088)
  (junction 2090) -> (junction 2089)
  (junction 2091) -> (junction 2090)
  (junction 2092) -> (junction 2091)
  (junction 2085) -> (junction 2093) : {
      |  SEND_RES_MESSAGE=!SEND_RES_MESSAGE;
      | }
  data: LENGTH_MESSAGE_CORRECT input boolean; CONDITION_CORRECT input boolean; REQ_SEQUENCE_OK input boolean; KEY_VALID input double; NEGATIVE_RES_CODE output uint8; PARAMETER_VALID input boolean; SUBFUNCTION_SUPPORTED_IN_ACTIVE_SESSION input boolean; SUBFUNCTION_SUPPORTED input boolean; SEND_RES_MESSAGE output boolean
